simulink model "SdbRxSigProc"  (R2022b)
settings: Solver=FixedStepDiscrete  StartTime=0.0  StopTime=10.0  FixedStep=0.05  RelTol=1e-3
(part 4 of 4)

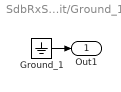
== system SdbRxSigProc/SdbRxSigProc_Init/Ground_1 ==
  Ground_1 : Ground
  Out1 : Outport  OutDataTypeStr=Bus: AmbTOutOfVeh  PortDimensions=1
  wires:
  Ground_1/1 -> Out1/1

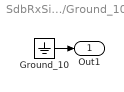
== system SdbRxSigProc/SdbRxSigProc_Init/Ground_10 ==
  Ground_10 : Ground
  Out1 : Outport  OutDataTypeStr=Bus: MemRecForCooltHeatrActvnCtrl  PortDimensions=1
  wires:
  Ground_10/1 -> Out1/1

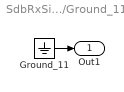
== system SdbRxSigProc/SdbRxSigProc_Init/Ground_11 ==
  Ground_11 : Ground
  Out1 : Outport  OutDataTypeStr=Bus: MemRecForCooltHeatrCtrl  PortDimensions=1
  wires:
  Ground_11/1 -> Out1/1

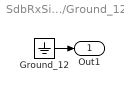
== system SdbRxSigProc/SdbRxSigProc_Init/Ground_12 ==
  Ground_12 : Ground
  Out1 : Outport  OutDataTypeStr=Bus: MemRecForFuTInFuHeatrMdl  PortDimensions=1
  wires:
  Ground_12/1 -> Out1/1

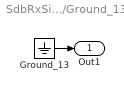
== system SdbRxSigProc/SdbRxSigProc_Init/Ground_13 ==
  Ground_13 : Ground
  Out1 : Outport  OutDataTypeStr=Bus: MemRecForHeatgInpMgr  PortDimensions=1
  wires:
  Ground_13/1 -> Out1/1

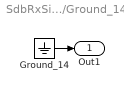
== system SdbRxSigProc/SdbRxSigProc_Init/Ground_14 ==
  Ground_14 : Ground
  Out1 : Outport  OutDataTypeStr=Bus: MemRecForSnsrMdlCooltT  PortDimensions=1
  wires:
  Ground_14/1 -> Out1/1

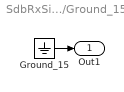
== system SdbRxSigProc/SdbRxSigProc_Init/Ground_15 ==
  Ground_15 : Ground
  Out1 : Outport  OutDataTypeStr=Bus: SunData  PortDimensions=1
  wires:
  Ground_15/1 -> Out1/1

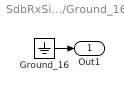
== system SdbRxSigProc/SdbRxSigProc_Init/Ground_16 ==
  Ground_16 : Ground
  Out1 : Outport  OutDataTypeStr=Bus: SunLoadOutOfVeh  PortDimensions=1
  wires:
  Ground_16/1 -> Out1/1

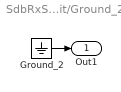
== system SdbRxSigProc/SdbRxSigProc_Init/Ground_2 ==
  Ground_2 : Ground
  Out1 : Outport  OutDataTypeStr=Bus: AmbTWithQly  PortDimensions=1
  wires:
  Ground_2/1 -> Out1/1

== system SdbRxSigProc/SdbRxSigProc_Init/Ground_3 ==
  Ground_3 : Ground
  Out1 : Outport  OutDataTypeStr=Bus: CmptmRelHum  PortDimensions=1
  wires:
  Ground_3/1 -> Out1/1

== system SdbRxSigProc/SdbRxSigProc_Init/Ground_4 ==
  Ground_4 : Ground
  Out1 : Outport  OutDataTypeStr=Bus: CooltHeatrAvlSts1  PortDimensions=1
  wires:
  Ground_4/1 -> Out1/1

== system SdbRxSigProc/SdbRxSigProc_Init/Ground_5 ==
  Ground_5 : Ground
  Out1 : Outport  OutDataTypeStr=Bus: EngT  PortDimensions=1
  wires:
  Ground_5/1 -> Out1/1

== system SdbRxSigProc/SdbRxSigProc_Init/Ground_6 ==
  Ground_6 : Ground
  Out1 : Outport  OutDataTypeStr=Bus: FogOutOfVeh  PortDimensions=1
  wires:
  Ground_6/1 -> Out1/1

== system SdbRxSigProc/SdbRxSigProc_Init/Ground_7 ==
  Ground_7 : Ground
  Out1 : Outport  OutDataTypeStr=Bus: HumOutOfVeh  PortDimensions=1
  wires:
  Ground_7/1 -> Out1/1

== system SdbRxSigProc/SdbRxSigProc_Init/Ground_8 ==
  Ground_8 : Ground
  Out1 : Outport  OutDataTypeStr=Bus: HvBattCoolgPwrHeatgMgrThermPred  PortDimensions=1
  wires:
  Ground_8/1 -> Out1/1

== system SdbRxSigProc/SdbRxSigProc_Runnable_sys/Ground_10 ==
  Ground_10 : Ground
  Out1 : Outport  OutDataTypeStr=Bus: DateTi30  PortDimensions=1
  wires:
  Ground_10/1 -> Out1/1

== system SdbRxSigProc/SdbRxSigProc_Runnable_sys/Ground_11 ==
  Ground_11 : Ground
  Out1 : Outport  OutDataTypeStr=Bus: DateTi30  PortDimensions=1
  wires:
  Ground_11/1 -> Out1/1

== system SdbRxSigProc/SdbRxSigProc_Runnable_sys/Ground_12 ==
  Ground_12 : Ground
  Out1 : Outport  OutDataTypeStr=Bus: DateTi30  PortDimensions=1
  wires:
  Ground_12/1 -> Out1/1

== system SdbRxSigProc/SdbRxSigProc_Runnable_sys/Ground_13 ==
  Ground_13 : Ground
  Out1 : Outport  OutDataTypeStr=Bus: DateTi30  PortDimensions=1
  wires:
  Ground_13/1 -> Out1/1

== system SdbRxSigProc/SdbRxSigProc_Runnable_sys/Ground_135 ==
  Ground_135 : Ground
  Out1 : Outport  OutDataTypeStr=Bus: SeatPosPercQF  PortDimensions=1
  wires:
  Ground_135/1 -> Out1/1

== system SdbRxSigProc/SdbRxSigProc_Runnable_sys/Ground_139 ==
  Ground_139 : Ground
  Out1 : Outport  OutDataTypeStr=Bus: Posn  PortDimensions=1
  wires:
  Ground_139/1 -> Out1/1

== system SdbRxSigProc/SdbRxSigProc_Runnable_sys/Ground_146 ==
  Ground_146 : Ground
  Out1 : Outport  OutDataTypeStr=Bus: RoadInclnRec1  PortDimensions=1
  wires:
  Ground_146/1 -> Out1/1

== system SdbRxSigProc/SdbRxSigProc_Runnable_sys/Ground_181 ==
  Ground_181 : Ground
  Out1 : Outport  OutDataTypeStr=Bus: DateTi30  PortDimensions=1
  wires:
  Ground_181/1 -> Out1/1

== system SdbRxSigProc/SdbRxSigProc_Runnable_sys/Ground_185 ==
  Ground_185 : Ground
  Out1 : Outport  OutDataTypeStr=Bus: WaterDepthInformation  PortDimensions=1
  wires:
  Ground_185/1 -> Out1/1

== system SdbRxSigProc/SdbRxSigProc_Runnable_sys/Ground_192 ==
  Ground_192 : Ground
  Out1 : Outport  OutDataTypeStr=Bus: AmbTOutOfVeh  PortDimensions=1
  wires:
  Ground_192/1 -> Out1/1

== system SdbRxSigProc/SdbRxSigProc_Runnable_sys/Ground_193 ==
  Ground_193 : Ground
  Out1 : Outport  OutDataTypeStr=Bus: AmbTWithQly  PortDimensions=1
  wires:
  Ground_193/1 -> Out1/1

== system SdbRxSigProc/SdbRxSigProc_Runnable_sys/Ground_195 ==
  Ground_195 : Ground
  Out1 : Outport  OutDataTypeStr=Bus: BltLockStFrnt  PortDimensions=1
  wires:
  Ground_195/1 -> Out1/1

== system SdbRxSigProc/SdbRxSigProc_Runnable_sys/Ground_196 ==
  Ground_196 : Ground
  Out1 : Outport  OutDataTypeStr=Bus: BltLockStFrnt  PortDimensions=1
  wires:
  Ground_196/1 -> Out1/1

== system SdbRxSigProc/SdbRxSigProc_Runnable_sys/Ground_197 ==
  Ground_197 : Ground
  Out1 : Outport  OutDataTypeStr=Bus: BltLockStRe  PortDimensions=1
  wires:
  Ground_197/1 -> Out1/1

== system SdbRxSigProc/SdbRxSigProc_Runnable_sys/Ground_198 ==
  Ground_198 : Ground
  Out1 : Outport  OutDataTypeStr=Bus: BltLockStRe  PortDimensions=1
  wires:
  Ground_198/1 -> Out1/1

== system SdbRxSigProc/SdbRxSigProc_Runnable_sys/Ground_199 ==
  Ground_199 : Ground
  Out1 : Outport  OutDataTypeStr=Bus: BltLockStRe  PortDimensions=1
  wires:
  Ground_199/1 -> Out1/1

== system SdbRxSigProc/SdbRxSigProc_Runnable_sys/Ground_200 ==
  Ground_200 : Ground
  Out1 : Outport  OutDataTypeStr=Bus: BltLockStRe  PortDimensions=1
  wires:
  Ground_200/1 -> Out1/1

== system SdbRxSigProc/SdbRxSigProc_Runnable_sys/Ground_201 ==
  Ground_201 : Ground
  Out1 : Outport  OutDataTypeStr=Bus: BltLockStRe  PortDimensions=1
  wires:
  Ground_201/1 -> Out1/1

== system SdbRxSigProc/SdbRxSigProc_Runnable_sys/Ground_21 ==
  Ground_21 : Ground
  Out1 : Outport  OutDataTypeStr=Bus: AMBStsgrp  PortDimensions=1
  wires:
  Ground_21/1 -> Out1/1

== system SdbRxSigProc/SdbRxSigProc_Runnable_sys/Ground_217 ==
  Ground_217 : Ground
  Out1 : Outport  OutDataTypeStr=Bus: CmptmRelHum  PortDimensions=1
  wires:
  Ground_217/1 -> Out1/1

== system SdbRxSigProc/SdbRxSigProc_Runnable_sys/Ground_218 ==
  Ground_218 : Ground
  Out1 : Outport  OutDataTypeStr=Bus: CoolgLoad1  PortDimensions=1
  wires:
  Ground_218/1 -> Out1/1

== system SdbRxSigProc/SdbRxSigProc_Runnable_sys/Ground_221 ==
  Ground_221 : Ground
  Out1 : Outport  OutDataTypeStr=Bus: CooltHeatrAvlSts1  PortDimensions=1
  wires:
  Ground_221/1 -> Out1/1

== system SdbRxSigProc/SdbRxSigProc_Runnable_sys/Ground_226 ==
  Ground_226 : Ground
  Out1 : Outport  OutDataTypeStr=Bus: DefrstSts  PortDimensions=1
  wires:
  Ground_226/1 -> Out1/1

== system SdbRxSigProc/SdbRxSigProc_Runnable_sys/Ground_232 ==
  Ground_232 : Ground
  Out1 : Outport  OutDataTypeStr=Bus: DrvrAirCdnrSetg  PortDimensions=1
  wires:
  Ground_232/1 -> Out1/1

== system SdbRxSigProc/SdbRxSigProc_Runnable_sys/Ground_241 ==
  Ground_241 : Ground
  Out1 : Outport  OutDataTypeStr=Bus: EngT  PortDimensions=1
  wires:
  Ground_241/1 -> Out1/1

== system SdbRxSigProc/SdbRxSigProc_Runnable_sys/Ground_242 ==
  Ground_242 : Ground
  Out1 : Outport  OutDataTypeStr=Bus: FogOutOfVeh  PortDimensions=1
  wires:
  Ground_242/1 -> Out1/1

== system SdbRxSigProc/SdbRxSigProc_Runnable_sys/Ground_258 ==
  Ground_258 : Ground
  Out1 : Outport  OutDataTypeStr=Bus: HumOutOfVeh  PortDimensions=1
  wires:
  Ground_258/1 -> Out1/1

== system SdbRxSigProc/SdbRxSigProc_Runnable_sys/Ground_263 ==
  Ground_263 : Ground
  Out1 : Outport  OutDataTypeStr=Bus: HvBattCoolgPwrHeatgMgrThermPred  PortDimensions=1
  wires:
  Ground_263/1 -> Out1/1

== system SdbRxSigProc/SdbRxSigProc_Runnable_sys/Ground_273 ==
  Ground_273 : Ground
  Out1 : Outport  OutDataTypeStr=Bus: LockgCenSts3  PortDimensions=1
  wires:
  Ground_273/1 -> Out1/1

== system SdbRxSigProc/SdbRxSigProc_Runnable_sys/Ground_279 ==
  Ground_279 : Ground
  Out1 : Outport  OutDataTypeStr=Bus: MemRecForCooltHeatrActvnCtrl  PortDimensions=1
  wires:
  Ground_279/1 -> Out1/1

== system SdbRxSigProc/SdbRxSigProc_Runnable_sys/Ground_280 ==
  Ground_280 : Ground
  Out1 : Outport  OutDataTypeStr=Bus: MemRecForCooltHeatrCtrl  PortDimensions=1
  wires:
  Ground_280/1 -> Out1/1

== system SdbRxSigProc/SdbRxSigProc_Runnable_sys/Ground_281 ==
  Ground_281 : Ground
  Out1 : Outport  OutDataTypeStr=Bus: MemRecForFuTInFuHeatrMdl  PortDimensions=1
  wires:
  Ground_281/1 -> Out1/1

== system SdbRxSigProc/SdbRxSigProc_Runnable_sys/Ground_282 ==
  Ground_282 : Ground
  Out1 : Outport  OutDataTypeStr=Bus: MemRecForHeatgInpMgr  PortDimensions=1
  wires:
  Ground_282/1 -> Out1/1

== system SdbRxSigProc/SdbRxSigProc_Runnable_sys/Ground_283 ==
  Ground_283 : Ground
  Out1 : Outport  OutDataTypeStr=Bus: MemRecForSnsrMdlCooltT  PortDimensions=1
  wires:
  Ground_283/1 -> Out1/1

== system SdbRxSigProc/SdbRxSigProc_Runnable_sys/Ground_29 ==
  Ground_29 : Ground
  Out1 : Outport  OutDataTypeStr=Bus: NavDir  PortDimensions=1
  wires:
  Ground_29/1 -> Out1/1

== system SdbRxSigProc/SdbRxSigProc_Runnable_sys/Ground_306 ==
  Ground_306 : Ground
  Out1 : Outport  OutDataTypeStr=Bus: RemStrHvCtrlReq  PortDimensions=1
  wires:
  Ground_306/1 -> Out1/1

== system SdbRxSigProc/SdbRxSigProc_Runnable_sys/Ground_326 ==
  Ground_326 : Ground
  Out1 : Outport  OutDataTypeStr=Bus: SunData  PortDimensions=1
  wires:
  Ground_326/1 -> Out1/1

== system SdbRxSigProc/SdbRxSigProc_Runnable_sys/Ground_327 ==
  Ground_327 : Ground
  Out1 : Outport  OutDataTypeStr=Bus: SunLoadOutOfVeh  PortDimensions=1
  wires:
  Ground_327/1 -> Out1/1

== system SdbRxSigProc/SdbRxSigProc_Runnable_sys/Ground_332 ==
  Ground_332 : Ground
  Out1 : Outport  OutDataTypeStr=Bus: VehModMngtGlbSafe1  PortDimensions=1
  wires:
  Ground_332/1 -> Out1/1

== system SdbRxSigProc/SdbRxSigProc_Runnable_sys/Ground_333 ==
  Ground_333 : Ground
  Out1 : Outport  OutDataTypeStr=Bus: VehSpdLgtSafe1  PortDimensions=1
  wires:
  Ground_333/1 -> Out1/1

== system SdbRxSigProc/SdbRxSigProc_Runnable_sys/Ground_339 ==
  Ground_339 : Ground
  Out1 : Outport  OutDataTypeStr=Bus: WipgInfo  PortDimensions=1
  wires:
  Ground_339/1 -> Out1/1

== system SdbRxSigProc/SdbRxSigProc_Runnable_sys/Ground_47 ==
  Ground_47 : Ground
  Out1 : Outport  OutDataTypeStr=Bus: SeatPosPercQF  PortDimensions=1
  wires:
  Ground_47/1 -> Out1/1

== system SdbRxSigProc/SdbRxSigProc_Runnable_sys/Ground_50 ==
  Ground_50 : Ground
  Out1 : Outport  OutDataTypeStr=Bus: ValveSts  PortDimensions=1
  wires:
  Ground_50/1 -> Out1/1

== system SdbRxSigProc/SdbRxSigProc_Runnable_sys/Ground_51 ==
  Ground_51 : Ground
  Out1 : Outport  OutDataTypeStr=Bus: FloatDrvingActv  PortDimensions=1
  wires:
  Ground_51/1 -> Out1/1

== system SdbRxSigProc/SdbRxSigProc_Runnable_sys/Ground_66 ==
  Ground_66 : Ground
  Out1 : Outport  OutDataTypeStr=Bus: GazeDirOri  PortDimensions=1
  wires:
  Ground_66/1 -> Out1/1

== system SdbRxSigProc/SdbRxSigProc_Runnable_sys/Ground_69 ==
  Ground_69 : Ground
  Out1 : Outport  OutDataTypeStr=Bus: PosIndct  PortDimensions=1
  wires:
  Ground_69/1 -> Out1/1

== system SdbRxSigProc/SdbRxSigProc_Runnable_sys/Ground_72 ==
  Ground_72 : Ground
  Out1 : Outport  OutDataTypeStr=Bus: DirModReq1  PortDimensions=1
  wires:
  Ground_72/1 -> Out1/1

== system SdbRxSigProc/SdbRxSigProc_Runnable_sys/Ground_80 ==
  Ground_80 : Ground
  Out1 : Outport  OutDataTypeStr=Bus: HmiFragraTypRatReq  PortDimensions=1
  wires:
  Ground_80/1 -> Out1/1

== system SdbRxSigProc/SdbRxSigProc_Runnable_sys/Ground_86 ==
  Ground_86 : Ground
  Out1 : Outport  OutDataTypeStr=Bus: GazeDirOri  PortDimensions=1
  wires:
  Ground_86/1 -> Out1/1

== system SdbRxSigProc/SdbRxSigProc_Runnable_sys/Ground_9 ==
  Ground_9 : Ground
  Out1 : Outport  OutDataTypeStr=Bus: DateTi30  PortDimensions=1
  wires:
  Ground_9/1 -> Out1/1
